simulink model "med_16a_2x_r113c"  (Simulink 7.4) (continued)
== system med_16a_2x_r113c == (continued)
      mask prompts: io_dir "I/O direction"; arith_type "Data Type"; bitwidth "Data bitwidth"; bin_pt "Data binary point"; sample_period "Sample period"
      mask init:
      | gw_name = clear_name(gcb);
      | config.source = str2func('software_register_init');
      | config.toplevel = gcb;
      | xBlock( config, {io_dir, arith_type, bitwidth, bin_pt, ... 
      |     sample_period, gw_name, latency});
      mask StyleString: popup(From Processor|To Processor),popup(Unsigned|Signed  (2's comp)),edit,edit,edit,edit
  gbe_tx_err_cnt0 : Subsystem  Tag=xps:sw_reg  mask{io_dir=To Processor, arith_type=Unsigned, bitwidth=32, bin_pt=0, sample_period=1, latency=0}
      mask prompts: io_dir "I/O direction"; arith_type "Data Type"; bitwidth "Data bitwidth"; bin_pt "Data binary point"; sample_period "Sample period"
      mask init:
      | gw_name = clear_name(gcb);
      | config.source = str2func('software_register_init');
      | config.toplevel = gcb;
      | xBlock( config, {io_dir, arith_type, bitwidth, bin_pt, ... 
      |     sample_period, gw_name, latency});
      mask StyleString: popup(From Processor|To Processor),popup(Unsigned|Signed  (2's comp)),edit,edit,edit,edit
  led0 : xps_library/gpio  Tag=xps:gpio  SystemSampleTime=-1  io_group=ROACH:gpioa  io_dir=out  arith_type=Boolean  bitwidth=1  bin_pt=0  bit_index=0  sample_period=1  use_single_ended=off  use_ddr=off  reg_iob=off  reg_clk_phase=0  termination=None
      link override convert: arith_type=Boolean  n_bits=1  bin_pt=0
  led1 : xps_library/gpio  Tag=xps:gpio  SystemSampleTime=-1  io_group=ROACH:gpioa  io_dir=out  arith_type=Boolean  bitwidth=1  bin_pt=0  bit_index=1  sample_period=1  use_single_ended=off  use_ddr=off  reg_iob=off  reg_clk_phase=0  termination=None
      link override convert: arith_type=Boolean  n_bits=1  bin_pt=0
  led2 : xps_library/gpio  Tag=xps:gpio  SystemSampleTime=-1  io_group=ROACH:gpioa  io_dir=out  arith_type=Boolean  bitwidth=1  bin_pt=0  bit_index=2  sample_period=1  use_single_ended=off  use_ddr=off  reg_iob=off  reg_clk_phase=0  termination=None
      link override convert: arith_type=Boolean  n_bits=1  bin_pt=0
  led3 : xps_library/gpio  Tag=xps:gpio  SystemSampleTime=-1  io_group=ROACH:gpioa  io_dir=out  arith_type=Boolean  bitwidth=1  bin_pt=0  bit_index=3  sample_period=1  use_single_ended=off  use_ddr=off  reg_iob=off  reg_clk_phase=0  termination=None
      link override convert: arith_type=Boolean  n_bits=1  bin_pt=0
  led4 : xps_library/gpio  Tag=xps:gpio  SystemSampleTime=-1  io_group=ROACH:gpioa  io_dir=out  arith_type=Boolean  bitwidth=1  bin_pt=0  bit_index=4  sample_period=1  use_single_ended=off  use_ddr=off  reg_iob=off  reg_clk_phase=0  termination=None
      link override convert: arith_type=Boolean  n_bits=1  bin_pt=0
  led5 : xps_library/gpio  Tag=xps:gpio  SystemSampleTime=-1  io_group=ROACH:gpioa  io_dir=out  arith_type=Boolean  bitwidth=1  bin_pt=0  bit_index=5  sample_period=1  use_single_ended=off  use_ddr=off  reg_iob=off  reg_clk_phase=0  termination=None
      link override convert: arith_type=Boolean  n_bits=1  bin_pt=0
  led6 : xps_library/gpio  Tag=xps:gpio  SystemSampleTime=-1  io_group=ROACH:gpioa  io_dir=out  arith_type=Boolean  bitwidth=1  bin_pt=0  bit_index=6  sample_period=1  use_single_ended=off  use_ddr=off  reg_iob=off  reg_clk_phase=0  termination=None
      link override convert: arith_type=Boolean  n_bits=1  bin_pt=0
  led7 : xps_library/gpio  Tag=xps:gpio  SystemSampleTime=-1  io_group=ROACH:gpioa  io_dir=out  arith_type=Boolean  bitwidth=1  bin_pt=0  bit_index=7  sample_period=1  use_single_ended=off  use_ddr=off  reg_iob=off  reg_clk_phase=0  termination=None
      link override convert: arith_type=Boolean  n_bits=1  bin_pt=0
  master_reset_sim1 : Constant  Value=0  OutDataType=sfix(16)  OutScaling=2^0
  master_reset_sim4 : Constant  Value=2  OutDataType=sfix(16)  OutScaling=2^0
  mux_idle_clk : Subsystem  Tag=xps:sw_reg  mask{io_dir=From Processor, arith_type=Unsigned, bitwidth=32, bin_pt=0, sample_period=1, latency=0}
      mask prompts: io_dir "I/O direction"; arith_type "Data Type"; bitwidth "Data bitwidth"; bin_pt "Data binary point"; sample_period "Sample period"
      mask init:
      | gw_name = clear_name(gcb);
      | config.source = str2func('software_register_init');
      | config.toplevel = gcb;
      | xBlock( config, {io_dir, arith_type, bitwidth, bin_pt, ... 
      |     sample_period, gw_name, latency});
      mask StyleString: popup(From Processor|To Processor),popup(Unsigned|Signed  (2's comp)),edit,edit,edit,edit
  mux_out : Subsystem
  p_eof_cnt : Subsystem  Tag=xps:sw_reg  mask{io_dir=To Processor, arith_type=Unsigned, bitwidth=32, bin_pt=0, sample_period=1, latency=0}
      mask prompts: io_dir "I/O direction"; arith_type "Data Type"; bitwidth "Data bitwidth"; bin_pt "Data binary point"; sample_period "Sample period"
      mask init:
      | gw_name = clear_name(gcb);
      | config.source = str2func('software_register_init');
      | config.toplevel = gcb;
      | xBlock( config, {io_dir, arith_type, bitwidth, bin_pt, ... 
      |     sample_period, gw_name, latency});
      mask StyleString: popup(From Processor|To Processor),popup(Unsigned|Signed  (2's comp)),edit,edit,edit,edit
  p_err_cnt : Subsystem  Tag=xps:sw_reg  mask{io_dir=To Processor, arith_type=Unsigned, bitwidth=32, bin_pt=0, sample_period=1, latency=0}
      mask prompts: io_dir "I/O direction"; arith_type "Data Type"; bitwidth "Data bitwidth"; bin_pt "Data binary point"; sample_period "Sample period"
      mask init:
      | gw_name = clear_name(gcb);
      | config.source = str2func('software_register_init');
      | config.toplevel = gcb;
      | xBlock( config, {io_dir, arith_type, bitwidth, bin_pt, ... 
      |     sample_period, gw_name, latency});
      mask StyleString: popup(From Processor|To Processor),popup(Unsigned|Signed  (2's comp)),edit,edit,edit,edit
  p_pkt_rdy_cnt : Subsystem  Tag=xps:sw_reg  mask{io_dir=To Processor, arith_type=Unsigned, bitwidth=32, bin_pt=0, sample_period=1, latency=0}
      mask prompts: io_dir "I/O direction"; arith_type "Data Type"; bitwidth "Data bitwidth"; bin_pt "Data binary point"; sample_period "Sample period"
      mask init:
      | gw_name = clear_name(gcb);
      | config.source = str2func('software_register_init');
      | config.toplevel = gcb;
      | xBlock( config, {io_dir, arith_type, bitwidth, bin_pt, ... 
      |     sample_period, gw_name, latency});
      mask StyleString: popup(From Processor|To Processor),popup(Unsigned|Signed  (2's comp)),edit,edit,edit,edit
  p_rb_done_cnt : Subsystem  Tag=xps:sw_reg  mask{io_dir=To Processor, arith_type=Unsigned, bitwidth=32, bin_pt=0, sample_period=1, latency=0}
      mask prompts: io_dir "I/O direction"; arith_type "Data Type"; bitwidth "Data bitwidth"; bin_pt "Data binary point"; sample_period "Sample period"
      mask init:
      | gw_name = clear_name(gcb);
      | config.source = str2func('software_register_init');
      | config.toplevel = gcb;
      | xBlock( config, {io_dir, arith_type, bitwidth, bin_pt, ... 
      |     sample_period, gw_name, latency});
      mask StyleString: popup(From Processor|To Processor),popup(Unsigned|Signed  (2's comp)),edit,edit,edit,edit
  p_valid_in_cnt : Subsystem  Tag=xps:sw_reg  mask{io_dir=To Processor, arith_type=Unsigned, bitwidth=32, bin_pt=0, sample_period=1, latency=0}
      mask prompts: io_dir "I/O direction"; arith_type "Data Type"; bitwidth "Data bitwidth"; bin_pt "Data binary point"; sample_period "Sample period"
      mask init:
      | gw_name = clear_name(gcb);
      | config.source = str2func('software_register_init');
      | config.toplevel = gcb;
      | xBlock( config, {io_dir, arith_type, bitwidth, bin_pt, ... 
      |     sample_period, gw_name, latency});
      mask StyleString: popup(From Processor|To Processor),popup(Unsigned|Signed  (2's comp)),edit,edit,edit,edit
  p_valid_out_cnt : Subsystem  Tag=xps:sw_reg  mask{io_dir=To Processor, arith_type=Unsigned, bitwidth=32, bin_pt=0, sample_period=1, latency=0}
      mask prompts: io_dir "I/O direction"; arith_type "Data Type"; bitwidth "Data bitwidth"; bin_pt "Data binary point"; sample_period "Sample period"
      mask init:
      | gw_name = clear_name(gcb);
      | config.source = str2func('software_register_init');
      | config.toplevel = gcb;
      | xBlock( config, {io_dir, arith_type, bitwidth, bin_pt, ... 
      |     sample_period, gw_name, latency});
      mask StyleString: popup(From Processor|To Processor),popup(Unsigned|Signed  (2's comp)),edit,edit,edit,edit
  pack_out0 : Subsystem  mask(pkt_pack_mask){vector_len=17*16/2*4*1024/2*2, heap_len=17*16/2*4*1024/2*2*2*4, fifo_latency=2, spead_msw=64, spead_lsw=40, spead_item=6144, pkt_bits=9}
      mask prompts: vector_len "vector length"; heap_len "System heap size"; fifo_latency "Fifo Latency"; spead_msw "SPEAD flavour (MSw)"; spead_lsw "SPEAD flavour (LSw)"; spead_item "SPEAD item number"; pkt_bits "desired payload length (2^?) 64-bit words"
      mask help:
      | This block generates packets from a datastream.
      |
      | Packets are created with a fixed header followed by a user-specified number of 64-bit options.
      |
      | Requires a 64-bit data stream.
      |
      | See the documentation for further details.
      mask init:
      | vector_bits = ceil(log2(vector_len));
      | pkt_len=2^(pkt_bits);
  pack_out1 : Subsystem  mask(pkt_pack_mask){vector_len=17*16/2*4*1024/2*2, heap_len=17*16/2*4*1024/2*2*2*4, fifo_latency=2, spead_msw=64, spead_lsw=40, spead_item=6144, pkt_bits=9}
      mask prompts: vector_len "vector length"; heap_len "System heap size"; fifo_latency "Fifo Latency"; spead_msw "SPEAD flavour (MSw)"; spead_lsw "SPEAD flavour (LSw)"; spead_item "SPEAD item number"; pkt_bits "desired payload length (2^?) 64-bit words"
      mask help:
      | This block generates packets from a datastream.
      |
      | Packets are created with a fixed header followed by a user-specified number of 64-bit options.
      |
      | Requires a 64-bit data stream.
      |
      | See the documentation for further details.
      mask init:
      | vector_bits = ceil(log2(vector_len));
      | pkt_len=2^(pkt_bits);
  pack_out_cnt0 : Subsystem  Tag=xps:sw_reg  mask{io_dir=To Processor, arith_type=Unsigned, bitwidth=32, bin_pt=0, sample_period=1, latency=0}
      mask prompts: io_dir "I/O direction"; arith_type "Data Type"; bitwidth "Data bitwidth"; bin_pt "Data binary point"; sample_period "Sample period"
      mask init:
      | gw_name = clear_name(gcb);
      | config.source = str2func('software_register_init');
      | config.toplevel = gcb;
      | xBlock( config, {io_dir, arith_type, bitwidth, bin_pt, ... 
      |     sample_period, gw_name, latency});
      mask StyleString: popup(From Processor|To Processor),popup(Unsigned|Signed  (2's comp)),edit,edit,edit,edit
  pack_out_cnt1 : Subsystem  Tag=xps:sw_reg  mask{io_dir=To Processor, arith_type=Unsigned, bitwidth=32, bin_pt=0, sample_period=1, latency=0}
      mask prompts: io_dir "I/O direction"; arith_type "Data Type"; bitwidth "Data bitwidth"; bin_pt "Data binary point"; sample_period "Sample period"
      mask init:
      | gw_name = clear_name(gcb);
      | config.source = str2func('software_register_init');
      | config.toplevel = gcb;
      | xBlock( config, {io_dir, arith_type, bitwidth, bin_pt, ... 
      |     sample_period, gw_name, latency});
      mask StyleString: popup(From Processor|To Processor),popup(Unsigned|Signed  (2's comp)),edit,edit,edit,edit
  pack_out_err0 : Subsystem  Tag=xps:sw_reg  mask{io_dir=To Processor, arith_type=Unsigned, bitwidth=32, bin_pt=0, sample_period=1, latency=0}
      mask prompts: io_dir "I/O direction"; arith_type "Data Type"; bitwidth "Data bitwidth"; bin_pt "Data binary point"; sample_period "Sample period"
      mask init:
      | gw_name = clear_name(gcb);
      | config.source = str2func('software_register_init');
      | config.toplevel = gcb;
      | xBlock( config, {io_dir, arith_type, bitwidth, bin_pt, ... 
      |     sample_period, gw_name, latency});
      mask StyleString: popup(From Processor|To Processor),popup(Unsigned|Signed  (2's comp)),edit,edit,edit,edit
  pack_out_err1 : Subsystem  Tag=xps:sw_reg  mask{io_dir=To Processor, arith_type=Unsigned, bitwidth=32, bin_pt=0, sample_period=1, latency=0}
      mask prompts: io_dir "I/O direction"; arith_type "Data Type"; bitwidth "Data bitwidth"; bin_pt "Data binary point"; sample_period "Sample period"
      mask init:
      | gw_name = clear_name(gcb);
      | config.source = str2func('software_register_init');
      | config.toplevel = gcb;
      | xBlock( config, {io_dir, arith_type, bitwidth, bin_pt, ... 
      |     sample_period, gw_name, latency});
      mask StyleString: popup(From Processor|To Processor),popup(Unsigned|Signed  (2's comp)),edit,edit,edit,edit
  posedge2 : casper_library_misc/posedge  Tag=Rising Edge Detector  SystemSampleTime=-1
  pulse_ext1 : Subsystem  mask{pulse_len=2^23}
      mask prompts: pulse_len "Length of Pulse"
      mask init:
      | bits = ceil(log2(pulse_len));
  pulse_ext2 : Subsystem  mask{pulse_len=2^23}
      mask prompts: pulse_len "Length of Pulse"
      mask init:
      | bits = ceil(log2(pulse_len));
  pulse_ext3 : Subsystem  mask{pulse_len=2^23}
      mask prompts: pulse_len "Length of Pulse"
      mask init:
      | bits = ceil(log2(pulse_len));
  pulse_ext4 : Subsystem  mask{pulse_len=2^23}
      mask prompts: pulse_len "Length of Pulse"
      mask init:
      | bits = ceil(log2(pulse_len));
  pulse_ext5 : Subsystem  mask{pulse_len=2^23}
      mask prompts: pulse_len "Length of Pulse"
      mask init:
      | bits = ceil(log2(pulse_len));
  pulse_ext6 : Subsystem  mask{pulse_len=2^23}
      mask prompts: pulse_len "Length of Pulse"
      mask init:
      | bits = ceil(log2(pulse_len));
  pulse_ext7 : Subsystem  mask{pulse_len=2^23}
      mask prompts: pulse_len "Length of Pulse"
      mask init:
      | bits = ceil(log2(pulse_len));
  pulse_ext8 : Subsystem  mask{pulse_len=2^23}
      mask prompts: pulse_len "Length of Pulse"
      mask init:
      | bits = ceil(log2(pulse_len));
  snap_pack0 : Subsystem  mask(snap_circ){nsamples=11}
      mask prompts: nsamples "Number of Samples (2^?)"
      mask help:
      | Backwards compatible with the normal snap block, but with the following upgrades:
      |  1) Ability to offset the trigger. Optionally delay the fabric trigger by a user-specified number of valids.
      |  2) 'Addr' register's MSb now indicates 'busy capturing'.
      |  3) Ability to capture continuously until a stop pulse is received. Data is captured into BRAM continuously, with old data being overwritten until a stop command is received. Then stop capturing when memory is filled. This enables you to get some data before and some data after the stop pulse. Use the 'tr_en_cnt' register to determine where you are in your vector.
  snap_tge0 : Subsystem  mask(snap_circ){nsamples=11}
      mask prompts: nsamples "Number of Samples (2^?)"
      mask help:
      | Backwards compatible with the normal snap block, but with the following upgrades:
      |  1) Ability to offset the trigger. Optionally delay the fabric trigger by a user-specified number of valids.
      |  2) 'Addr' register's MSb now indicates 'busy capturing'.
      |  3) Ability to capture continuously until a stop pulse is received. Data is captured into BRAM continuously, with old data being overwritten until a stop command is received. Then stop capturing when memory is filled. This enables you to get some data before and some data after the stop pulse. Use the 'tr_en_cnt' register to determine where you are in your vector.
  snap_vacc0 : Subsystem  mask(snap_circ){nsamples=11}
      mask prompts: nsamples "Number of Samples (2^?)"
      mask help:
      | Backwards compatible with the normal snap block, but with the following upgrades:
      |  1) Ability to offset the trigger. Optionally delay the fabric trigger by a user-specified number of valids.
      |  2) 'Addr' register's MSb now indicates 'busy capturing'.
      |  3) Ability to capture continuously until a stop pulse is received. Data is captured into BRAM continuously, with old data being overwritten until a stop command is received. Then stop capturing when memory is filled. This enables you to get some data before and some data after the stop pulse. Use the 'tr_en_cnt' register to determine where you are in your vector.
  snap_xaui0 : Subsystem  mask{nsamples=13}
      mask prompts: nsamples "Number of Samples (2^?)"
  snap_xeng0 : Subsystem  mask(snap_circ){nsamples=11}
      mask prompts: nsamples "Number of Samples (2^?)"
      mask help:
      | Backwards compatible with the normal snap block, but with the following upgrades:
      |  1) Ability to offset the trigger. Optionally delay the fabric trigger by a user-specified number of valids.
      |  2) 'Addr' register's MSb now indicates 'busy capturing'.
      |  3) Ability to capture continuously until a stop pulse is received. Data is captured into BRAM continuously, with old data being overwritten until a stop command is received. Then stop capturing when memory is filled. This enables you to get some data before and some data after the stop pulse. Use the 'tr_en_cnt' register to determine where you are in your vector.
  tge_out : xps_library/ten_Gbe_v2  Tag=xps:tengbe_v2  SystemSampleTime=-1  port=1  rx_dist_ram=on  large_frames=off  show_param=on  pre_emph=3  swing=800  fab_en=off  fab_mac=hex2dec('123456780000')  fab_ip=192*(2^24) + 168*(2^16) + 5*(2^8) + 20*(2^0)  fab_udp=10000  fab_gate=1  cpu_rx_en=on  cpu_tx_en=on
  tvg_sel : Subsystem  Tag=xps:sw_reg  mask{io_dir=From Processor, arith_type=Unsigned, bitwidth=32, bin_pt=0, sample_period=1, latency=0}
      mask prompts: io_dir "I/O direction"; arith_type "Data Type"; bitwidth "Data bitwidth"; bin_pt "Data binary point"; sample_period "Sample period"
      mask init:
      | gw_name = clear_name(gcb);
      | config.source = str2func('software_register_init');
      | config.toplevel = gcb;
      | xBlock( config, {io_dir, arith_type, bitwidth, bin_pt, ... 
      |     sample_period, gw_name, latency});
      mask StyleString: popup(From Processor|To Processor),popup(Unsigned|Signed  (2's comp)),edit,edit,edit,edit
  vacc0 : Subsystem  mask{ants_bits=4, freq_bits=10, xeng_bits=1, which_qdr=qdr0}
      mask prompts: ants_bits "Number of antennas (2^n)"; freq_bits "Number of frequency channels (2^n)"; xeng_bits "Number of X engines (2^n)"; which_qdr "QDR"
      mask help: Hardcoded for X engines with a demux of 8!
      mask init:
      | vector_len = (2^ants_bits)*(2^ants_bits +1) /2 *8 * 2^(freq_bits-xeng_bits);
      | set_param([gcb, '/qv'], 'which_qdr', which_qdr);
      | fmtstr = sprintf('Vector Length: %d', vector_len);
      | set_param(gcb, 'AttributesFormatString', fmtstr);
      settings: AttributesFormatString=Vector Length: 557056
      mask StyleString: edit,edit,edit,popup(qdr0|qdr1)
  vacc1 : Subsystem  mask{ants_bits=4, freq_bits=10, xeng_bits=1, which_qdr=qdr1}
      mask prompts: ants_bits "Number of antennas (2^n)"; freq_bits "Number of frequency channels (2^n)"; xeng_bits "Number of X engines (2^n)"; which_qdr "QDR"
      mask help: Hardcoded for X engines with a demux of 8!
      mask init: same as med_16a_2x_r113c/vacc0
      settings: AttributesFormatString=Vector Length: 557056
      mask StyleString: edit,edit,edit,popup(qdr0|qdr1)
  vacc_cnt0 : Subsystem  Tag=xps:sw_reg  mask{io_dir=To Processor, arith_type=Unsigned, bitwidth=32, bin_pt=0, sample_period=1, latency=0}
      mask prompts: io_dir "I/O direction"; arith_type "Data Type"; bitwidth "Data bitwidth"; bin_pt "Data binary point"; sample_period "Sample period"
      mask init:
      | gw_name = clear_name(gcb);
      | config.source = str2func('software_register_init');
      | config.toplevel = gcb;
      | xBlock( config, {io_dir, arith_type, bitwidth, bin_pt, ... 
      |     sample_period, gw_name, latency});
      mask StyleString: popup(From Processor|To Processor),popup(Unsigned|Signed  (2's comp)),edit,edit,edit,edit
  vacc_cnt1 : Subsystem  Tag=xps:sw_reg  mask{io_dir=To Processor, arith_type=Unsigned, bitwidth=32, bin_pt=0, sample_period=1, latency=0}
      mask prompts: io_dir "I/O direction"; arith_type "Data Type"; bitwidth "Data bitwidth"; bin_pt "Data binary point"; sample_period "Sample period"
      mask init:
      | gw_name = clear_name(gcb);
      | config.source = str2func('software_register_init');
      | config.toplevel = gcb;
      | xBlock( config, {io_dir, arith_type, bitwidth, bin_pt, ... 
      |     sample_period, gw_name, latency});
      mask StyleString: popup(From Processor|To Processor),popup(Unsigned|Signed  (2's comp)),edit,edit,edit,edit
  vacc_err_cnt0 : Subsystem  Tag=xps:sw_reg  mask{io_dir=To Processor, arith_type=Unsigned, bitwidth=32, bin_pt=0, sample_period=1, latency=0}
      mask prompts: io_dir "I/O direction"; arith_type "Data Type"; bitwidth "Data bitwidth"; bin_pt "Data binary point"; sample_period "Sample period"
      mask init:
      | gw_name = clear_name(gcb);
      | config.source = str2func('software_register_init');
      | config.toplevel = gcb;
      | xBlock( config, {io_dir, arith_type, bitwidth, bin_pt, ... 
      |     sample_period, gw_name, latency});
      mask StyleString: popup(From Processor|To Processor),popup(Unsigned|Signed  (2's comp)),edit,edit,edit,edit
  vacc_err_cnt1 : Subsystem  Tag=xps:sw_reg  mask{io_dir=To Processor, arith_type=Unsigned, bitwidth=32, bin_pt=0, sample_period=1, latency=0}
      mask prompts: io_dir "I/O direction"; arith_type "Data Type"; bitwidth "Data bitwidth"; bin_pt "Data binary point"; sample_period "Sample period"
      mask init:
      | gw_name = clear_name(gcb);
      | config.source = str2func('software_register_init');
      | config.toplevel = gcb;
      | xBlock( config, {io_dir, arith_type, bitwidth, bin_pt, ... 
      |     sample_period, gw_name, latency});
      mask StyleString: popup(From Processor|To Processor),popup(Unsigned|Signed  (2's comp)),edit,edit,edit,edit
  vacc_ld_status0 : Subsystem  Tag=xps:sw_reg  mask{io_dir=To Processor, arith_type=Unsigned, bitwidth=32, bin_pt=0, sample_period=1, latency=0}
      mask prompts: io_dir "I/O direction"; arith_type "Data Type"; bitwidth "Data bitwidth"; bin_pt "Data binary point"; sample_period "Sample period"
      mask init:
      | gw_name = clear_name(gcb);
      | config.source = str2func('software_register_init');
      | config.toplevel = gcb;
      | xBlock( config, {io_dir, arith_type, bitwidth, bin_pt, ... 
      |     sample_period, gw_name, latency});
      mask StyleString: popup(From Processor|To Processor),popup(Unsigned|Signed  (2's comp)),edit,edit,edit,edit
  vacc_ld_status1 : Subsystem  Tag=xps:sw_reg  mask{io_dir=To Processor, arith_type=Unsigned, bitwidth=32, bin_pt=0, sample_period=1, latency=0}
      mask prompts: io_dir "I/O direction"; arith_type "Data Type"; bitwidth "Data bitwidth"; bin_pt "Data binary point"; sample_period "Sample period"
      mask init:
      | gw_name = clear_name(gcb);
      | config.source = str2func('software_register_init');
      | config.toplevel = gcb;
      | xBlock( config, {io_dir, arith_type, bitwidth, bin_pt, ... 
      |     sample_period, gw_name, latency});
      mask StyleString: popup(From Processor|To Processor),popup(Unsigned|Signed  (2's comp)),edit,edit,edit,edit
  vacc_monitor : Subsystem
  vacc_monitor1 : Subsystem
  vacc_monitor2 : Subsystem
  vacc_monitor3 : Subsystem
  vacc_monitor4 : Subsystem
  vacc_monitor5 : Subsystem
  vacc_monitor6 : Subsystem
  vacc_monitor7 : Subsystem
  vacc_sync_cnt : Subsystem  Tag=xps:sw_reg  mask{io_dir=To Processor, arith_type=Unsigned, bitwidth=32, bin_pt=0, sample_period=1, latency=0}
      mask prompts: io_dir "I/O direction"; arith_type "Data Type"; bitwidth "Data bitwidth"; bin_pt "Data binary point"; sample_period "Sample period"
      mask init:
      | gw_name = clear_name(gcb);
      | config.source = str2func('software_register_init');
      | config.toplevel = gcb;
      | xBlock( config, {io_dir, arith_type, bitwidth, bin_pt, ... 
      |     sample_period, gw_name, latency});
      mask StyleString: popup(From Processor|To Processor),popup(Unsigned|Signed  (2's comp)),edit,edit,edit,edit
  vacc_time_lsw : Subsystem  Tag=xps:sw_reg  mask{io_dir=From Processor, arith_type=Unsigned, bitwidth=32, bin_pt=0, sample_period=1, latency=0}
      mask prompts: io_dir "I/O direction"; arith_type "Data Type"; bitwidth "Data bitwidth"; bin_pt "Data binary point"; sample_period "Sample period"
      mask init:
      | gw_name = clear_name(gcb);
      | config.source = str2func('software_register_init');
      | config.toplevel = gcb;
      | xBlock( config, {io_dir, arith_type, bitwidth, bin_pt, ... 
      |     sample_period, gw_name, latency});
      mask StyleString: popup(From Processor|To Processor),popup(Unsigned|Signed  (2's comp)),edit,edit,edit,edit
  vacc_time_msw : Subsystem  Tag=xps:sw_reg  mask{io_dir=From Processor, arith_type=Unsigned, bitwidth=32, bin_pt=0, sample_period=1, latency=0}
      mask prompts: io_dir "I/O direction"; arith_type "Data Type"; bitwidth "Data bitwidth"; bin_pt "Data binary point"; sample_period "Sample period"
      mask init:
      | gw_name = clear_name(gcb);
      | config.source = str2func('software_register_init');
      | config.toplevel = gcb;
      | xBlock( config, {io_dir, arith_type, bitwidth, bin_pt, ... 
      |     sample_period, gw_name, latency});
      mask StyleString: popup(From Processor|To Processor),popup(Unsigned|Signed  (2's comp)),edit,edit,edit,edit
  vacc_tvg1 : Subsystem  mask{len=16*17/2*1024/2*4*2}
      mask prompts: len "Number of Vectors"
      mask init:
      | fmtstr = sprintf('Vector Length: %d', len);
      | set_param(gcb, 'AttributesFormatString', fmtstr);
      settings: AttributesFormatString=Vector Length: 557056
  vacc_tvg2 : Subsystem  mask{len=16*17/2*1024/2*4*2}
      mask prompts: len "Number of Vectors"
      mask init:
      | fmtstr = sprintf('Vector Length: %d', len);
      | set_param(gcb, 'AttributesFormatString', fmtstr);
      settings: AttributesFormatString=Vector Length: 557056
  vacc_vld_cnt : Subsystem  Tag=xps:sw_reg  mask{io_dir=To Processor, arith_type=Unsigned, bitwidth=32, bin_pt=0, sample_period=1, latency=0}
      mask prompts: io_dir "I/O direction"; arith_type "Data Type"; bitwidth "Data bitwidth"; bin_pt "Data binary point"; sample_period "Sample period"
      mask init:
      | gw_name = clear_name(gcb);
      | config.source = str2func('software_register_init');
      | config.toplevel = gcb;
      | xBlock( config, {io_dir, arith_type, bitwidth, bin_pt, ... 
      |     sample_period, gw_name, latency});
      mask StyleString: popup(From Processor|To Processor),popup(Unsigned|Signed  (2's comp)),edit,edit,edit,edit
  xaui_buf0 : Subsystem
  xaui_cnt0 : Subsystem  Tag=xps:sw_reg  mask{io_dir=To Processor, arith_type=Unsigned, bitwidth=32, bin_pt=0, sample_period=1, latency=0}
      mask prompts: io_dir "I/O direction"; arith_type "Data Type"; bitwidth "Data bitwidth"; bin_pt "Data binary point"; sample_period "Sample period"
      mask init:
      | gw_name = clear_name(gcb);
      | config.source = str2func('software_register_init');
      | config.toplevel = gcb;
      | xBlock( config, {io_dir, arith_type, bitwidth, bin_pt, ... 
      |     sample_period, gw_name, latency});
      mask StyleString: popup(From Processor|To Processor),popup(Unsigned|Signed  (2's comp)),edit,edit,edit,edit
  xaui_err0 : Subsystem  Tag=xps:sw_reg  mask{io_dir=To Processor, arith_type=Unsigned, bitwidth=32, bin_pt=0, sample_period=1, latency=0}
      mask prompts: io_dir "I/O direction"; arith_type "Data Type"; bitwidth "Data bitwidth"; bin_pt "Data binary point"; sample_period "Sample period"
      mask init:
      | gw_name = clear_name(gcb);
      | config.source = str2func('software_register_init');
      | config.toplevel = gcb;
      | xBlock( config, {io_dir, arith_type, bitwidth, bin_pt, ... 
      |     sample_period, gw_name, latency});
      mask StyleString: popup(From Processor|To Processor),popup(Unsigned|Signed  (2's comp)),edit,edit,edit,edit
  xaui_pack0 : Subsystem  mask{payload_len=32, xeng_bits=1, skip_xeng_bits=1, skip_ant_bits=4}
      mask prompts: payload_len "Payload Length (in 64 bit words)"; xeng_bits "Number of X engines (2^?)"; skip_xeng_bits "X engines per IP address (2^?)"; skip_ant_bits "Antennas per XAUI link (2^?)"
      mask help: Packetizes data coming in over a XAUI interface. A packet consists of a 64 bit header (48 bits of "mcnt" and 16 bits of antenna), followed by 64 x "payload" bits of payload. "Mcnt" (master count) is a counter which keeps track of how many packets have been transmitted since the last "mrst".
      mask init:
      | packet_len = payload_len + 1;
      | packet_bits = ceil(log2(packet_len));
      | payload_bits = ceil(log2(payload_len));
  xaui_sync_mcnt0 : Subsystem  Tag=xps:sw_reg  mask{io_dir=To Processor, arith_type=Unsigned, bitwidth=32, bin_pt=0, sample_period=1, latency=0}
      mask prompts: io_dir "I/O direction"; arith_type "Data Type"; bitwidth "Data bitwidth"; bin_pt "Data binary point"; sample_period "Sample period"
      mask init:
      | gw_name = clear_name(gcb);
      | config.source = str2func('software_register_init');
      | config.toplevel = gcb;
      | xBlock( config, {io_dir, arith_type, bitwidth, bin_pt, ... 
      |     sample_period, gw_name, latency});
      mask StyleString: popup(From Processor|To Processor),popup(Unsigned|Signed  (2's comp)),edit,edit,edit,edit
  xeng0 : Subsystem  AncestorBlock=casper_library_correlator/xeng  mask(xeng){n_ants=16, n_bits=4, acc_len=128, demux_factor=8, add_latency=1, mult_latency=2, bram_latency=2, use_ded_mult=1, use_bram_delay=1}
      mask prompts: n_ants "Number of antennas"; n_bits "Bit-width of samples in"; acc_len "Accumulation length"; add_latency "Adder latency"; mult_latency "Multiplier latency"; bram_latency "BRAM latency"; use_ded_mult "Implementation: Multiplier type (0=slices 1=embedded 2=bram)"; use_bram_delay "Implementation: Delay type (0=SLR, 1=BRAM)"
      mask help: CASPER X engine with added internal valid data masking functionality, descramble and integral demux. Based on Aaron Parsons' and Lynn Urry's original X engine design.
      mask init:
      | xeng_init(gcb, ...
      |     'n_ants', n_ants, ...
      |     'n_bits', n_bits, ...
      |     'acc_len', acc_len, ...
      |     'demux_factor', demux_factor, ...
      |     'add_latency', add_latency, ...
      |     'mult_latency', mult_latency, ...
      |     'bram_latency', bram_latency, ...
      |     'use_ded_mult', use_ded_mult, ...
      |     'use_bram_delay', use_bram_delay);
      settings: AttributesFormatString=n_ant=16, bits=4, mult=1, bram=1
      mask StyleString: edit,edit,edit,popup(1|2|4|8),edit,edit,edit,edit,edit
  xeng1 : Subsystem  AncestorBlock=casper_library_correlator/xeng  mask(xeng){n_ants=16, n_bits=4, acc_len=128, demux_factor=8, add_latency=1, mult_latency=2, bram_latency=2, use_ded_mult=1, use_bram_delay=1}
      mask prompts: n_ants "Number of antennas"; n_bits "Bit-width of samples in"; acc_len "Accumulation length"; add_latency "Adder latency"; mult_latency "Multiplier latency"; bram_latency "BRAM latency"; use_ded_mult "Implementation: Multiplier type (0=slices 1=embedded 2=bram)"; use_bram_delay "Implementation: Delay type (0=SLR, 1=BRAM)"
      mask help: CASPER X engine with added internal valid data masking functionality, descramble and integral demux. Based on Aaron Parsons' and Lynn Urry's original X engine design.
      mask init: same as med_16a_2x_r113c/xeng0
      settings: AttributesFormatString=n_ant=16, bits=4, mult=1, bram=1
      mask StyleString: edit,edit,edit,popup(1|2|4|8),edit,edit,edit,edit,edit
  xeng_sync_cnt : Subsystem  Tag=xps:sw_reg  mask{io_dir=To Processor, arith_type=Unsigned, bitwidth=32, bin_pt=0, sample_period=1, latency=0}
      mask prompts: io_dir "I/O direction"; arith_type "Data Type"; bitwidth "Data bitwidth"; bin_pt "Data binary point"; sample_period "Sample period"
      mask init:
      | gw_name = clear_name(gcb);
      | config.source = str2func('software_register_init');
      | config.toplevel = gcb;
      | xBlock( config, {io_dir, arith_type, bitwidth, bin_pt, ... 
      |     sample_period, gw_name, latency});
      mask StyleString: popup(From Processor|To Processor),popup(Unsigned|Signed  (2's comp)),edit,edit,edit,edit
  xeng_tvg0 : Subsystem  AncestorBlock=casper_library/Correlator/xeng_tvg  mask{ant_bits=4, x_int_bits=7, sync_period=20}
      mask prompts: ant_bits "Number of Antennas (2^?):"; x_int_bits "X Integration Length (2^?):"; sync_period "Sync Pulse Period (2^?):"
  xeng_tvg1 : Subsystem  AncestorBlock=casper_library/Correlator/xeng_tvg  mask{ant_bits=4, x_int_bits=7, sync_period=20}
      mask prompts: ant_bits "Number of Antennas (2^?):"; x_int_bits "X Integration Length (2^?):"; sync_period "Sync Pulse Period (2^?):"
  xeng_vld_cnt : Subsystem  Tag=xps:sw_reg  mask{io_dir=To Processor, arith_type=Unsigned, bitwidth=32, bin_pt=0, sample_period=1, latency=0}
      mask prompts: io_dir "I/O direction"; arith_type "Data Type"; bitwidth "Data bitwidth"; bin_pt "Data binary point"; sample_period "Sample period"
      mask init:
      | gw_name = clear_name(gcb);
      | config.source = str2func('software_register_init');
      | config.toplevel = gcb;
      | xBlock( config, {io_dir, arith_type, bitwidth, bin_pt, ... 
      |     sample_period, gw_name, latency});
      mask StyleString: popup(From Processor|To Processor),popup(Unsigned|Signed  (2's comp)),edit,edit,edit,edit
  wires:
  Constant10/1 -> XAUI0/1
  Constant9/1 -> XAUI0/2
  Constant1/1 -> XAUI0/4
  Constant2/1 -> XAUI0/5
  Constant3/1 -> XAUI0/3
  Delay2/1(q) -> [xaui0_valid]
  Delay1/1(q) -> [xaui0_oob]
  Delay54/1(q) -> [xaui0_data]
  Delay3/1(q) -> [xaui0_link_dn]
  XAUI0/1 -> Delay54/1(d)
  XAUI0/2 -> Delay1/1(d)
  XAUI0/3 -> Terminator4/1
  XAUI0/4 -> Delay2/1(d)
  XAUI0/5 -> Delay3/1(d)
  XAUI0/6 -> Terminator1/1
  XAUI0/7 -> Terminator2/1
  demux_gbe0/1(valid) -> buffer4/2(vld)
  demux_gbe1/1(valid) -> buffer1/2(vld)
  [buf0_data] -> buffer4/1(din)
  [buf0_ant] -> buffer4/6(ant)
  [buf0_eof] -> buffer4/3(eof)
  [buf0_we] -> demux_gbe0/1(we)
  [buf0_mcnt] -> demux_gbe0/2(mcnt), buffer4/4(mcnt)
  xeng_tvg0/1(sync_out) -> vacc_monitor7/1(sync), xeng0/1(sync_in)
  xeng_tvg0/2(data_out) -> xeng0/2(ant)
  xeng_tvg0/3(valid_out) -> vacc_monitor7/2(valid), xeng0/3(window_valid)
  Delay18/1(q) -> Delay14/1(d)
  xeng_tvg1/1(sync_out) -> xeng1/1(sync_in)
  xeng_tvg1/2(data_out) -> xeng1/2(ant)
  xeng_tvg1/3(valid_out) -> xeng1/3(window_valid)
  [xeng_tvg_sel] -> xeng_tvg1/1(tvg_sel)
  Delay19/1(q) -> xeng1/4(mcnt_in)
  Delay20/1(q) -> xeng_tvg1/2(sync)
  Delay21/1(q) -> xeng_tvg1/3(data_in)
  Delay22/1(q) -> xeng_tvg1/4(valid_in)
  Delay23/1(q) -> Delay19/1(d)
  xeng0/1(sync_out) -> Delay6/1
  xeng0/3(valid) -> Delay5/1
  [vacc_tvg_sel] -> vacc_tvg1/1(tvg_sel)
  Constant12/1 -> snap_xeng0/4(stop)
  16b_32b1/1(Out1) -> vacc_tvg1/3(data_in), snap_xeng0/1(data_in)
  xeng0/2(acc) -> Delay4/1
  xeng1/1(sync_out) -> Delay51/1
  xeng1/3(valid) -> Delay/1
  [vacc_tvg_sel] -> vacc_tvg2/1(tvg_sel)
  16b_32b2/1(Out1) -> vacc_tvg2/3(data_in)
  xeng1/2(acc) -> Delay50/1
  Subsystem9/1(data_out) -> snap_xaui0/1(data_in)
  Subsystem11/1(tx_cnt) -> Delay68/1(d)
  [cnt_rst] -> Subsystem11/1(rst)
  Delay24/1(q) -> xaui_pack0/1(din)
  Delay28/1(q) -> xaui_pack0/2(oob)
  Delay29/1(q) -> xaui_pack0/3(valid_in)
  Delay30/1(q) -> xaui_pack0/4(linkdown_in)
  Subsystem11/2(err_cnt) -> Delay69/1(d)
  [xaui_pkt_err] -> Subsystem11/5(pkt_err)
  xaui_cnt0/1(sim_reg_out) -> Terminator21/1
  xaui_err0/1(sim_reg_out) -> Terminator22/1
  Delay68/1(q) -> xaui_cnt0/1(reg_out)
  Delay69/1(q) -> xaui_err0/1(reg_out)
  xaui_sync_mcnt0/1(sim_reg_out) -> Terminator15/1
  Delay93/1(q) -> xaui_sync_mcnt0/1(reg_out)
  Subsystem11/3(mcnt_at_sync) -> Delay93/1(d)
  Subsystem9/2(oob_out) -> snap_xaui0/2(oob_in)
  Subsystem9/3(we_out) -> snap_xaui0/3(we)
  Subsystem9/4(trig_out) -> snap_xaui0/4(trig)
  Subsystem9/5(stop) -> snap_xaui0/5(stop)
  [xaui0_data] -> Subsystem11/2(data)
  [xaui0_oob] -> Subsystem11/3(oob)
  [xaui0_valid] -> Subsystem11/4(valid)
  [xaui0_data] -> Delay24/1(d)
  [xaui0_oob] -> Delay28/1(d)
  [xaui0_valid] -> Delay29/1(d)
  [xaui0_link_dn] -> Delay30/1(d)
  [xaui0_data] -> Subsystem9/1(data_in)
  [xaui0_oob] -> Subsystem9/2(oob_in)
  [xaui0_valid] -> Subsystem9/3(we_in)
  [xaui0_link_dn] -> Subsystem9/4(link_down)
  Pulse Generator2/1 -> ctrl/1(sim_reg_in)
  ctrl/1(reg_in) -> Delay25/1(d)
  dev_sel3/1 -> Delay88/1(d)
  Slice7/1 -> posedge2/1
  posedge2/1 -> Delay58/1(d)
  Delay25/1(q) -> dev_sel3/1, Slice7/1, Slice8/1, Slice14/1
  Delay58/1(q) -> [cnt_rst]
  Delay88/1(q) -> [vacc_rst]
  master_reset_sim4/1 -> acc_len/1(sim_reg_in)
  acc_len/1(reg_in) -> Delay26/1(d)
  Delay26/1(q) -> [acc_len]
  tvg_sel/1(reg_in) -> Delay89/1(d)
  Delay89/1(q) -> dev_sel5/1, Slice5/1
  dev_sel5/1 -> [xeng_tvg_sel]
  Slice5/1 -> [vacc_tvg_sel]
  master_reset_sim1/1 -> tvg_sel/1(sim_reg_in)
  Delay33/1(q) -> [feng_sync]
  Delay34/1(q) -> [xaui_link_down]
  Delay35/1(q) -> [xaui_pkt_err]
  Delay36/1(q) -> [feng_mrst]
  Delay37/1(q) -> [xaui_pkt_data]
  Delay32/1(q) -> [xaui_pkt_valid]
  Delay31/1(q) -> [xaui_pkt_eof]
  xaui_pack0/1(dout) -> Delay37/1(d)
  xaui_pack0/2(valid_out) -> Delay32/1(d)
  xaui_pack0/3(eof) -> Delay31/1(d)
  xaui_pack0/4(discard) -> Delay35/1(d)
  xaui_pack0/5(feng_sync) -> Delay33/1(d)
  xaui_pack0/6(linkdown_out) -> Delay34/1(d)
  xaui_pack0/7(mrst) -> Delay36/1(d)
  xaui_pack0/8(hdr) -> Delay27/1(d)
  xaui_pack0/9(hdr_valid) -> Delay38/1(d)
  Delay27/1(q) -> [xaui_pkt_hdr]
  Delay38/1(q) -> [xaui_hdr_valid]
  [xaui_pkt_hdr] -> xaui_buf0/5(hdr_in)
  xaui_buf0/4(mcnt) -> [buf0_mcnt]
  xaui_buf0/5(ant) -> [buf0_ant]
  xaui_buf0/6(flag) -> [buf0_flag]
  [xaui_pkt_eof] -> xaui_buf0/4(eof_in)
  xaui_buf0/3(eof_out) -> [buf0_eof]
  [xaui_pkt_valid] -> xaui_buf0/2(valid)
  xaui_buf0/2(we) -> [buf0_we]
  xaui_buf0/1(dout) -> [buf0_data]
  [xaui_pkt_data] -> xaui_buf0/1(din)
  [xaui_hdr_valid] -> xaui_buf0/3(hdr_valid)
  [buf0_mcnt] -> demux_gbe1/2(mcnt), buffer1/4(mcnt)
  [buf0_data] -> buffer1/1(din)
  [buf0_we] -> demux_gbe1/1(we)
  [buf0_eof] -> buffer1/3(eof)
  [buf0_ant] -> buffer1/6(ant)
  err_clear_sim1/1 -> gbe_out_port/1(sim_reg_in)
  gbe_out_port/1(reg_in) -> Delay41/1(d)
  Delay41/1(q) -> [gbe_out_txport]
  err_clear_sim5/1 -> gbe_out_ip/1(sim_reg_in)
  gbe_out_ip/1(reg_in) -> Delay40/1(d)
  Delay40/1(q) -> [gbe_out_ip_addr]
  err_clear_sim3/1 -> board_id/1(sim_reg_in)
  board_id/1(reg_in) -> Delay72/1(d)
  Delay72/1(q) -> [board_id]
  Slice14/1 -> Delay100/1(d)
  Delay100/1(q) -> [gbe_out_enable]
  Delay122/1(q) -> [gbe_out_rst]
  Slice8/1 -> Delay122/1(d)
  Constant/1 -> [id1]
  Constant4/1 -> [id0]
  vacc_tvg1/1(sync_out) -> vacc0/5(sync_in), vacc_monitor6/1(sync)
  vacc_tvg1/2(data_out) -> vacc0/6(data_in)
  vacc_tvg1/3(valid_out) -> vacc0/7(we), vacc_monitor6/2(valid)
  delay5/1 -> vacc0/8(pcnt_in)
  xeng0/4(mcnt_out) -> delay5/1
  [vacc_rst] -> vacc0/1(rst)
  Constant5/1 -> vacc_time_msw/1(sim_reg_in)
  Constant6/1 -> vacc_time_lsw/1(sim_reg_in)
  vacc_time_msw/1(reg_in) -> Slice4/1, Slice13/1
  Slice13/1 -> Concat12/1
  vacc_time_lsw/1(reg_in) -> Concat12/2
  Slice4/1 -> [vacc_ld_arm]
  Concat12/1 -> [vacc_ld_time]
  [vacc_ld_arm] -> vacc0/2(arm)
  [vacc_ld_time] -> vacc0/3(load_time)
  [acc_len] -> vacc0/4(acc_len)
  vacc_monitor/2(spec_cnt) -> vacc_cnt0/1(reg_out)
  vacc_monitor/1(error_cnt) -> vacc_err_cnt0/1(reg_out)
  [cnt_rst] -> vacc_monitor/1(cnt_rst)
  [new_acc0] -> vacc_monitor/2(new_acc)
  [vacc_err0] -> vacc_monitor/3(error)
  [vacc_ld_stat0] -> vacc_ld_status0/1(reg_out)
  [cnt_rst] -> vacc_monitor2/1(cnt_rst)
  vacc_monitor2/1(pkt_cnt) -> pack_out_cnt0/1(reg_out)
  vacc_monitor2/2(error_cnt) -> pack_out_err0/1(reg_out)
  [vacc_eof0] -> vacc_monitor2/2(eof)
  [pack_err0] -> vacc_monitor2/3(error)
  [cnt_rst] -> vacc_monitor3/1(cnt_rst)
  vacc_monitor3/1(pkt_cnt) -> pack_out_cnt1/1(reg_out)
  vacc_monitor3/2(error_cnt) -> pack_out_err1/1(reg_out)
  [vacc_eof1] -> vacc_monitor3/2(eof)
  [pack_err1] -> vacc_monitor3/3(error)
  vacc_monitor1/2(spec_cnt) -> vacc_cnt1/1(reg_out)
  vacc_monitor1/1(error_cnt) -> vacc_err_cnt1/1(reg_out)
  [cnt_rst] -> vacc_monitor1/1(cnt_rst)
  [vacc_err1] -> vacc_monitor1/3(error)
  [new_acc1] -> vacc_monitor1/2(new_acc)
  [vacc_ld_stat1] -> vacc_ld_status1/1(reg_out)
  delay4/1 -> pack_out0/1(data_in), snap_vacc0/1(data_in)
  vacc0/2(data_out) -> delay4/1
  vacc0/4(valid_out) -> delay1/1, [valid_in0]
  delay1/1 -> pack_out0/2(valid_in), snap_vacc0/2(we)
  vacc0/6(rb_done) -> delay2/1, [rb_done0]
  vacc0/7(timestamp) -> delay3/1
  delay2/1 -> pack_out0/4(rb_done), snap_vacc0/3(trig)
  delay3/1 -> pack_out0/5(timestamp)
  [id0] -> pack_out0/6(xeng_id)
  vacc0/10(error_out) -> [vacc_err0]
  vacc0/8(ld_status) -> [vacc_ld_stat0]
  vacc0/1(new_acc) -> [new_acc0]
  vacc0/5(pkt_rdy) -> [vacc_pkt_rdy0]
  pack_out0/5(fetch) -> vacc0/9(re)
  Delay42/1(q) -> [vacc_dout0]
  Delay43/1(q) -> [vacc_valid0]
  Delay39/1(q) -> [vacc_eof0]
  Delay44/1(q) -> [pack_err0]
  pack_out0/1(data_out) -> Delay42/1(d), Slice/1
  pack_out0/2(valid_out) -> Delay43/1(d), snap_pack0/2(we)
  pack_out0/3(eof) -> Delay39/1(d), snap_pack0/3(trig)
  pack_out0/4(err) -> Delay44/1(d)
  [gbe_out_enable] -> Logical1/2
  Logical1/1 -> [gbe_txvld]
  [vacc_dout0] -> mux_out/1(din0)
  [vacc_valid0] -> mux_out/2(valid0)
  [vacc_eof0] -> mux_out/3(eof0)
  [vacc_pkt_rdy0] -> mux_out/4(data_rdy0)
  [vacc_dout1] -> mux_out/5(din1)
  [vacc_valid1] -> mux_out/6(valid1)
  [vacc_eof1] -> mux_out/7(eof1)
  [vacc_pkt_rdy1] -> mux_out/8(data_rdy1)
  mux_out/4(pkt_send0) -> [tx_pkt0]
  mux_out/5(pkt_send1) -> [tx_pkt1]
  mux_out/2(valid) -> Logical1/1, snap_tge0/2(we)
  mux_out/3(eof) -> [gbe_txeof]
  [vacc_rst] -> vacc1/1(rst)
  [vacc_ld_arm] -> vacc1/2(arm)
  [vacc_ld_time] -> vacc1/3(load_time)
  [acc_len] -> vacc1/4(acc_len)
  vacc_tvg2/1(sync_out) -> vacc1/5(sync_in)
  vacc_tvg2/2(data_out) -> vacc1/6(data_in)
  vacc_tvg2/3(valid_out) -> vacc1/7(we)
  xeng1/4(mcnt_out) -> delay6/1
  delay6/1 -> vacc1/8(pcnt_in)
  delay10/1 -> pack_out1/1(data_in)
  vacc1/2(data_out) -> delay10/1
  vacc1/4(valid_out) -> delay7/1
  delay7/1 -> pack_out1/2(valid_in)
  vacc1/6(rb_done) -> delay8/1
  vacc1/7(timestamp) -> delay9/1
  delay8/1 -> pack_out1/4(rb_done)
  delay9/1 -> pack_out1/5(timestamp)
  [id1] -> pack_out1/6(xeng_id)
  vacc1/1(new_acc) -> [new_acc1]
  vacc1/5(pkt_rdy) -> [vacc_pkt_rdy1]
  vacc1/8(ld_status) -> [vacc_ld_stat1]
  vacc1/10(error_out) -> [vacc_err1]
  Delay46/1(q) -> [vacc_dout1]
  Delay47/1(q) -> [vacc_valid1]
  Delay45/1(q) -> [vacc_eof1]
  Delay48/1(q) -> [pack_err1]
  pack_out1/1(data_out) -> Delay46/1(d)
  pack_out1/2(valid_out) -> Delay47/1(d)
  pack_out1/3(eof) -> Delay45/1(d)
  pack_out1/4(err) -> Delay48/1(d)
  pack_out1/5(fetch) -> vacc1/9(re)
  [tx_pkt0] -> pack_out0/3(pkt_rdy)
  [tx_pkt1] -> pack_out1/3(pkt_rdy)
  [vacc_err0] -> snap_vacc0/4(stop)
  [gbe_out_rst] -> delay12/1
  delay12/1 -> tge_out/1
  mux_out/1(dout) -> delay11/1, Slice2/1
  delay11/1 -> tge_out/2
  [gbe_txvld] -> delay13/1
  delay13/1 -> tge_out/3
  [gbe_out_ip_addr] -> delay14/1
  delay14/1 -> tge_out/4
  [gbe_out_txport] -> delay15/1
  delay15/1 -> tge_out/5
  [gbe_txeof] -> delay16/1
  delay16/1 -> tge_out/6
  delay17/1 -> tge_out/7
  delay18/1 -> tge_out/8
  Constant7/1 -> delay17/1, delay18/1
  gbe_err/1(tx_cnt) -> Delay63/1(d)
  [cnt_rst] -> gbe_err/1(rst)
  gbe_err/2(err_cnt) -> Delay64/1(d)
  Delay63/1(q) -> gbe_tx_cnt0/1(reg_out)
  Delay64/1(q) -> gbe_tx_err_cnt0/1(reg_out)
  [gbe_tx_over] -> gbe_err/4(overflow)
  [gbe_txeof] -> gbe_err/3(eof)
  [gbe_txvld] -> gbe_err/2(valid)
  tge_out/5 -> [gbe_tx_over]
  Constant8/1 -> snap_tge0/4(stop)
  [gbe_txeof] -> snap_tge0/3(trig)
  Constant11/1 -> snap_pack0/4(stop)
  Slice/1 -> snap_pack0/1(data_in)
  Slice2/1 -> snap_tge0/1(data_in)
  vacc_monitor4/1(valid_cnt) -> p_valid_out_cnt/1(reg_out)
  vacc_monitor4/2(eof_cnt) -> p_eof_cnt/1(reg_out)
  vacc_monitor4/3(err_cnt) -> p_err_cnt/1(reg_out)
  vacc_monitor4/4(valid_in_cnt) -> p_valid_in_cnt/1(reg_out)
  vacc_monitor4/5(pkt_rdy_cnt) -> p_pkt_rdy_cnt/1(reg_out)
  vacc_monitor4/6(rb_done_cnt) -> p_rb_done_cnt/1(reg_out)
  [cnt_rst] -> vacc_monitor4/1(cnt_rst)
  [vacc_eof0] -> vacc_monitor4/2(eof)
  [vacc_valid0] -> vacc_monitor4/3(valid)
  [vacc_err0] -> vacc_monitor4/4(err)
  [tx_pkt0] -> vacc_monitor4/6(pkt_rdy)
  [valid_in0] -> vacc_monitor4/5(valid_in)
  [rb_done0] -> vacc_monitor4/7(rb_done)
  vacc_monitor5/1(valid_cnt) -> g_valid_cnt/1(reg_out)
  vacc_monitor5/2(eof_cnt) -> g_eof_cnt/1(reg_out)
  vacc_monitor5/3(send0_cnt) -> g_send0_cnt/1(reg_out)
  vacc_monitor5/4(send1_cnt) -> g_send1_cnt/1(reg_out)
  [tx_pkt0] -> vacc_monitor5/4(send0)
  [tx_pkt1] -> vacc_monitor5/5(send1)
  [cnt_rst] -> vacc_monitor5/1(cnt_rst)
  [gbe_txeof] -> vacc_monitor5/2(eof)
  [gbe_txvld] -> vacc_monitor5/3(valid)
  Delay49/1(q) -> [flushing]
  pack_out0/6(flush) -> Delay49/1(d)
  [flushing] -> vacc_monitor5/6(flush)
  vacc_monitor5/5(flush_cnt) -> g_send1_cnt1/1(reg_out)
  Delay/1 -> vacc_tvg2/4(valid_in)
  Delay50/1 -> 16b_32b2/1(In1)
  Delay51/1 -> vacc_tvg2/2(sync_in)
  vacc_monitor6/1(sync_cnt) -> vacc_sync_cnt/1(reg_out)
  vacc_monitor6/2(vld_cnt) -> vacc_vld_cnt/1(reg_out)
  vacc_monitor7/1(sync_cnt) -> xeng_sync_cnt/1(reg_out)
  vacc_monitor7/2(vld_cnt) -> xeng_vld_cnt/1(reg_out)
  [feng_mrst] -> buffer4/5(mrst)
  [feng_mrst] -> buffer1/5(mrst)
  Delay6/1 -> snap_xeng0/3(trig), vacc_tvg1/2(sync_in)
  Delay4/1 -> 16b_32b1/1(In1)
  Delay5/1 -> snap_xeng0/2(we), vacc_tvg1/4(valid_in)
  [xeng_tvg_sel] -> xeng_tvg0/1(tvg_sel)
  Delay15/1(q) -> xeng_tvg0/2(sync)
  Delay16/1(q) -> xeng_tvg0/3(data_in)
  Delay17/1(q) -> xeng_tvg0/4(valid_in)
  err_clear_sim2/1 -> mux_idle_clk/1(sim_reg_in)
  mux_idle_clk/1(reg_in) -> Delay7/1(d)
  Delay7/1(q) -> [mux_idle]
  [mux_idle] -> mux_out/9(idle_rate)
  led0/1 -> Terminator39/1
  led1/1 -> Terminator3/1
  led2/1 -> Terminator6/1
  led3/1 -> Terminator5/1
  led4/1 -> Terminator8/1
  led5/1 -> Terminator7/1
  led6/1 -> Terminator10/1
  led7/1 -> Terminator9/1
  Delay118/1(q) -> pulse_ext2/1(in)
  [new_acc0] -> Logical2/1
  [new_acc1] -> Logical2/2
  Logical2/1 -> Delay118/1(d)
  pulse_ext2/1(out) -> led0/1
  tge_out/1 -> [gbe_tx_up]
  Delay8/1(q) -> pulse_ext4/1(in)
  [gbe_tx_up] -> Delay8/1(d)
  pulse_ext4/1(out) -> led1/1
  Delay9/1(q) -> pulse_ext1/1(in)
  [gbe_tx_over] -> Delay9/1(d)
  pulse_ext1/1(out) -> led2/1
  Delay10/1(q) -> pulse_ext3/1(in)
  [gbe_out_enable] -> Delay10/1(d)
  pulse_ext3/1(out) -> led3/1
  Delay96/1(q) -> pulse_ext5/1(in)
  Logical4/1 -> Delay96/1(d)
  [vacc_err0] -> Logical4/1
  [vacc_err1] -> Logical4/2
  pulse_ext5/1(out) -> led4/1
  Delay11/1(q) -> pulse_ext6/1(in)
  Logical3/1 -> Delay11/1(d)
  pulse_ext6/1(out) -> led5/1
  [pack_err0] -> Logical3/1
  [pack_err1] -> Logical3/2
  Delay12/1(q) -> pulse_ext7/1(in)
  pulse_ext7/1(out) -> led7/1
  [xaui_pkt_err] -> Delay12/1(d)
  Delay13/1(q) -> pulse_ext8/1(in)
  [xaui0_link_dn] -> Delay13/1(d)
  pulse_ext8/1(out) -> led6/1
  buffer1/4(mcnt_out) -> Delay23/1(d)
  buffer1/3(window_vld) -> Delay22/1(d)
  buffer1/2(dout) -> Delay21/1(d)
  buffer1/1(sync_out) -> Delay20/1(d)
  buffer4/4(mcnt_out) -> Delay18/1(d)
  buffer4/3(window_vld) -> Delay17/1(d)
  buffer4/2(dout) -> Delay16/1(d)
  buffer4/1(sync_out) -> Delay15/1(d)
  Delay14/1(q) -> xeng0/4(mcnt_in)
